simulink model "pasp_16i16c1ks"  (Simulink 7.3)
settings: Solver=ode45  StartTime=0.0  StopTime=10  RelTol=1e-3
undefined symbols (not defined in the file or in its folder): BEE2_ctrl, dist_gbe, ip_ctr, makereorderarray, pasp_lib, pfb_coeff_gen_calc
libraries: xbsIndex_r4 x2297, casper_library x261, xps_library x57, gavrt_library x2
(part 5 of 28)

== system pasp_16i16c1ks/pasp/scale_ctr ==
  Reset : Inport  OutDataType=sfix(16)  OutScaling=2^0
  Counter : xbsIndex_r4/Counter  cnt_type=Free Running  cnt_to=Inf  operation=Up  start_count=0  cnt_by_val=1  arith_type=Unsigned  n_bits=3  bin_pt=0  load_pin=off  rst=on  en=off  explicit_period=off  period=1  dbl_ovrd=off  use_behavioral_HDL=off  implementation=Fabric  use_rpm=on  block_type=counter
      settings: xl_area=[0, 0, 0, 0, 0, 0, 0]
  data_in : xbsIndex_r4/Constant  arith_type=Unsigned  const=1  n_bits=32  bin_pt=0  explicit_period=on  period=1  equ=P=C  opselect=C  inp2=PCIN>>17  opr=+  inp1=P  carry=CIN  dbl_ovrd=off  block_type=constant
  pol0_even_bram : xps_library/Shared BRAM  Tag=xps:bram  SystemSampleTime=-1  arith_type=Unsigned  addr_width=11  data_width=32  data_bin_pt=0  init_vals=1:8  sample_rate=1
      link override convert_din: arith_type=Unsigned
      link override mem/sim_data_in: arith_type=Unsigned
      link override pasp_16i16c128s_pasp_scale_ctr_pol0_even_bram_data_out: arith_type=Unsigned
  pol0_odd_bram : xps_library/Shared BRAM  Tag=xps:bram  SystemSampleTime=-1  arith_type=Unsigned  addr_width=11  data_width=32  data_bin_pt=0  init_vals=1:8  sample_rate=1
      link override convert_din: arith_type=Unsigned
      link override mem/sim_data_in: arith_type=Unsigned
      link override pasp_16i16c128s_pasp_scale_ctr_pol0_odd_bram_data_out: arith_type=Unsigned
  pol1_even_bram : xps_library/Shared BRAM  Tag=xps:bram  SystemSampleTime=-1  arith_type=Unsigned  addr_width=11  data_width=32  data_bin_pt=0  init_vals=1:8  sample_rate=1
      link override convert_din: arith_type=Unsigned
      link override mem/sim_data_in: arith_type=Unsigned
      link override pasp_16i16c128s_pasp_scale_ctr_pol1_even_bram_data_out: arith_type=Unsigned
  pol1_odd_bram : xps_library/Shared BRAM  Tag=xps:bram  SystemSampleTime=-1  arith_type=Unsigned  addr_width=11  data_width=32  data_bin_pt=0  init_vals=1:8  sample_rate=1
      link override convert_din: arith_type=Unsigned
      link override mem/sim_data_in: arith_type=Unsigned
      link override pasp_16i16c128s_pasp_scale_ctr_pol1_odd_bram_data_out: arith_type=Unsigned
  we : xbsIndex_r4/Constant  arith_type=Boolean  const=0  n_bits=1  bin_pt=0  explicit_period=on  period=1  equ=P=C  opselect=C  inp2=PCIN>>17  opr=+  inp1=P  carry=CIN  dbl_ovrd=off  block_type=constant
  Pol0_Odd : Outport  OutDataType=sfix(16)  OutScaling=2^0
  Pol0_Even : Outport  Port=2  OutDataType=sfix(16)  OutScaling=2^0
  Pol1_Odd : Outport  Port=3  OutDataType=sfix(16)  OutScaling=2^0
  Pol1_Even : Outport  Port=4  OutDataType=sfix(16)  OutScaling=2^0
  wires:
  Reset/1 -> Counter/1
  Counter/1 -> pol0_odd_bram/1, pol0_even_bram/1, pol1_odd_bram/1, pol1_even_bram/1
  data_in/1 -> pol0_odd_bram/2, pol0_even_bram/2, pol1_odd_bram/2, pol1_even_bram/2
  we/1 -> pol0_odd_bram/3, pol0_even_bram/3, pol1_odd_bram/3, pol1_even_bram/3
  pol0_odd_bram/1 -> Pol0_Odd/1
  pol0_even_bram/1 -> Pol0_Even/1
  pol1_odd_bram/1 -> Pol1_Odd/1
  pol1_even_bram/1 -> Pol1_Even/1

== system pasp_16i16c1ks/pasp/scope_output1 ==
  rst : Inport  OutDataType=sfix(16)  OutScaling=2^0
  en : Inport  Port=2  OutDataType=sfix(16)  OutScaling=2^0
  data_in : Inport  Port=3  OutDataType=sfix(16)  OutScaling=2^0
  bram : xps_library/Shared BRAM  Tag=xps:bram  SystemSampleTime=-1  arith_type="Signed  (2's comp)"  addr_width=11  data_width=32  data_bin_pt=17  init_vals=[1:2^11]  sample_rate=1
  freeze_cntr : casper_library/Misc/freeze_cntr  SystemSampleTime=-1  CounterBits=11
  wires:
  freeze_cntr/1 -> bram/1
  freeze_cntr/2 -> bram/3
  en/1 -> freeze_cntr/1
  data_in/1 -> bram/2
  rst/1 -> freeze_cntr/2

== system pasp_16i16c1ks/pasp/scope_output2 ==
  rst : Inport  OutDataType=sfix(16)  OutScaling=2^0
  en : Inport  Port=2  OutDataType=sfix(16)  OutScaling=2^0
  data_in : Inport  Port=3  OutDataType=sfix(16)  OutScaling=2^0
  bram : xps_library/Shared BRAM  Tag=xps:bram  SystemSampleTime=-1  arith_type="Signed  (2's comp)"  addr_width=11  data_width=32  data_bin_pt=17  init_vals=[1:2^11]  sample_rate=1
  freeze_cntr : casper_library/Misc/freeze_cntr  SystemSampleTime=-1  CounterBits=11
  wires:
  freeze_cntr/1 -> bram/1
  freeze_cntr/2 -> bram/3
  en/1 -> freeze_cntr/1
  data_in/1 -> bram/2
  rst/1 -> freeze_cntr/2

== system pasp_16i16c1ks/pasp/scope_output3 ==
  rst : Inport  OutDataType=sfix(16)  OutScaling=2^0
  en : Inport  Port=2  OutDataType=sfix(16)  OutScaling=2^0
  data_in : Inport  Port=3  OutDataType=sfix(16)  OutScaling=2^0
  bram : xps_library/Shared BRAM  Tag=xps:bram  SystemSampleTime=-1  arith_type="Signed  (2's comp)"  addr_width=11  data_width=32  data_bin_pt=17  init_vals=[1:2^11]  sample_rate=1
  freeze_cntr : casper_library/Misc/freeze_cntr  SystemSampleTime=-1  CounterBits=11
  wires:
  rst/1 -> freeze_cntr/2
  data_in/1 -> bram/2
  en/1 -> freeze_cntr/1
  freeze_cntr/2 -> bram/3
  freeze_cntr/1 -> bram/1

== system pasp_16i16c1ks/pasp/scope_output4 ==
  rst : Inport  OutDataType=sfix(16)  OutScaling=2^0
  en : Inport  Port=2  OutDataType=sfix(16)  OutScaling=2^0
  data_in : Inport  Port=3  OutDataType=sfix(16)  OutScaling=2^0
  bram : xps_library/Shared BRAM  Tag=xps:bram  SystemSampleTime=-1  arith_type="Signed  (2's comp)"  addr_width=11  data_width=32  data_bin_pt=17  init_vals=[1:2^11]  sample_rate=1
  freeze_cntr : casper_library/Misc/freeze_cntr  SystemSampleTime=-1  CounterBits=11
  wires:
  freeze_cntr/1 -> bram/1
  freeze_cntr/2 -> bram/3
  en/1 -> freeze_cntr/1
  data_in/1 -> bram/2
  rst/1 -> freeze_cntr/2

== system pasp_16i16c1ks/pasp/scope_output5 ==
  rst : Inport  OutDataType=sfix(16)  OutScaling=2^0
  en : Inport  Port=2  OutDataType=sfix(16)  OutScaling=2^0
  data_in : Inport  Port=3  OutDataType=sfix(16)  OutScaling=2^0
  bram : xps_library/Shared BRAM  Tag=xps:bram  SystemSampleTime=-1  arith_type="Signed  (2's comp)"  addr_width=11  data_width=32  data_bin_pt=17  init_vals=[1:2^11]  sample_rate=1
  freeze_cntr : casper_library/Misc/freeze_cntr  SystemSampleTime=-1  CounterBits=11
  wires:
  freeze_cntr/1 -> bram/1
  freeze_cntr/2 -> bram/3
  en/1 -> freeze_cntr/1
  data_in/1 -> bram/2
  rst/1 -> freeze_cntr/2

== system pasp_16i16c1ks/pasp/scope_output6 ==
  rst : Inport  OutDataType=sfix(16)  OutScaling=2^0
  en : Inport  Port=2  OutDataType=sfix(16)  OutScaling=2^0
  data_in : Inport  Port=3  OutDataType=sfix(16)  OutScaling=2^0
  bram : xps_library/Shared BRAM  Tag=xps:bram  SystemSampleTime=-1  arith_type="Signed  (2's comp)"  addr_width=11  data_width=32  data_bin_pt=17  init_vals=[1:2^11]  sample_rate=1
  freeze_cntr : casper_library/Misc/freeze_cntr  SystemSampleTime=-1  CounterBits=11
  wires:
  freeze_cntr/1 -> bram/1
  freeze_cntr/2 -> bram/3
  en/1 -> freeze_cntr/1
  data_in/1 -> bram/2
  rst/1 -> freeze_cntr/2

== system pasp_16i16c1ks/pasp/scope_output7 ==
  rst : Inport  OutDataType=sfix(16)  OutScaling=2^0
  en : Inport  Port=2  OutDataType=sfix(16)  OutScaling=2^0
  data_in : Inport  Port=3  OutDataType=sfix(16)  OutScaling=2^0
  bram : xps_library/Shared BRAM  Tag=xps:bram  SystemSampleTime=-1  arith_type="Signed  (2's comp)"  addr_width=11  data_width=32  data_bin_pt=17  init_vals=[1:2^11]  sample_rate=1
  freeze_cntr : casper_library/Misc/freeze_cntr  SystemSampleTime=-1  CounterBits=11
  wires:
  rst/1 -> freeze_cntr/2
  data_in/1 -> bram/2
  en/1 -> freeze_cntr/1
  freeze_cntr/2 -> bram/3
  freeze_cntr/1 -> bram/1

== system pasp_16i16c1ks/pasp/scope_output8 ==
  rst : Inport  OutDataType=sfix(16)  OutScaling=2^0
  en : Inport  Port=2  OutDataType=sfix(16)  OutScaling=2^0
  data_in : Inport  Port=3  OutDataType=sfix(16)  OutScaling=2^0
  bram : xps_library/Shared BRAM  Tag=xps:bram  SystemSampleTime=-1  arith_type="Signed  (2's comp)"  addr_width=11  data_width=32  data_bin_pt=17  init_vals=[1:2^11]  sample_rate=1
  freeze_cntr : casper_library/Misc/freeze_cntr  SystemSampleTime=-1  CounterBits=11
  wires:
  freeze_cntr/1 -> bram/1
  freeze_cntr/2 -> bram/3
  en/1 -> freeze_cntr/1
  data_in/1 -> bram/2
  rst/1 -> freeze_cntr/2

== system pasp_16i16c1ks/pasp/sync_generator ==
  period : Inport  OutDataType=sfix(16)  OutScaling=2^0
  reset : Inport  Port=2  OutDataType=sfix(16)  OutScaling=2^0
  Convert : xbsIndex_r4/Convert  arith_type=Boolean  n_bits=8  bin_pt=6  quantization=Truncate  overflow=Wrap  latency=1  dbl_ovrd=off  pipeline=off  block_type=convert
      settings: xl_area=[0, 0, 0, 0, 0, 0, 0]  block_version=10.1.3
  Counter : xbsIndex_r4/Counter  cnt_type=Free Running  cnt_to=Inf  operation=Up  start_count=1  cnt_by_val=1  arith_type=Unsigned  n_bits=32  bin_pt=0  load_pin=off  rst=on  en=off  explicit_period=on  period=1  dbl_ovrd=off  use_behavioral_HDL=off  implementation=Fabric  use_rpm=off  block_type=counter
  Logical : xbsIndex_r4/Logical  logical_function=OR  inputs=2  en=off  latency=0  precision=Full  arith_type=Unsigned  n_bits=8  bin_pt=2  align_bp=on  dbl_ovrd=off  block_type=logical
      settings: xl_area=[0, 0, 0, 0, 0, 0, 0]
  Relational : xbsIndex_r4/Relational  mode=a>=b  en=off  latency=1  dbl_ovrd=off  block_type=relational
      settings: xl_area=[0, 0, 0, 0, 0, 0, 0]  block_version=10.1.3
  cns : xbsIndex_r4/Constant  arith_type=Unsigned  const=4  n_bits=3  bin_pt=0  explicit_period=off  period=1  equ=P=C  opselect=C  inp2=PCIN>>17  opr=+  inp1=P  carry=CIN  dbl_ovrd=off  block_type=constant
  period_sub : xbsIndex_r4/AddSub  mode=Subtraction  use_carryin=off  use_carryout=off  en=off  latency=0  precision=User Defined  arith_type=Unsigned  n_bits=32  bin_pt=0  quantization=Truncate  overflow=Wrap  dbl_ovrd=off  use_behavioral_HDL=on  hw_selection=Fabric  pipelined=off  use_rpm=on  block_type=addsub
      settings: xl_area=[0, 0, 0, 0, 0, 0, 0]  block_version=10.1.3
  posedge : casper_library/Misc/posedge  Tag=Rising Edge Detector  SystemSampleTime=-1
  sync : Outport  OutDataType=sfix(16)  OutScaling=2^0
  wires:
  Counter/1 -> Relational/1
  Relational/1 -> Convert/1
  Convert/1 -> Logical/2
  Logical/1 -> posedge/1, Counter/1
  reset/1 -> Logical/1
  period/1 -> period_sub/1
  period_sub/1 -> Relational/2
  cns/1 -> period_sub/2
  posedge/1 -> sync/1

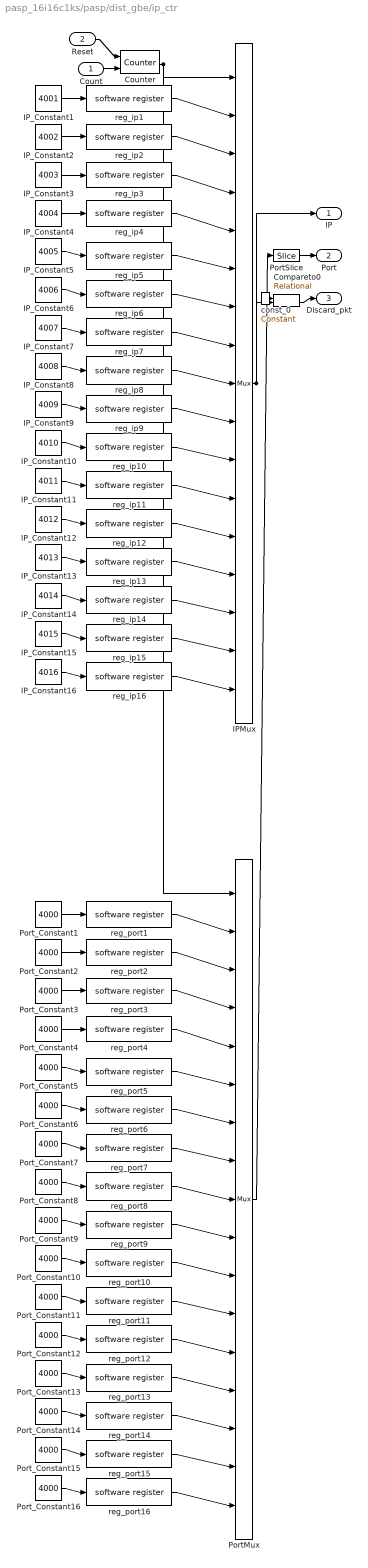
== system pasp_16i16c1ks/pasp/dist_gbe/ip_ctr ==
  Count : Inport
  Reset : Inport  Port=2  OutDataType=sfix(16)  OutScaling=2^0
  Compareto0 : xbsIndex_r4/Relational  mode=a=b  en=off  latency=1  dbl_ovrd=off  block_type=relational
  Counter : xbsIndex_r4/Counter  cnt_type=Free Running  cnt_to=Inf  operation=Up  start_count=0  cnt_by_val=1  arith_type=Unsigned  n_bits=4  bin_pt=0  load_pin=off  rst=on  en=on  explicit_period=on  period=1  dbl_ovrd=off  use_behavioral_HDL=off  implementation=Fabric  block_type=counter
      settings: block_version=11.4
  IPMux : xbsIndex_r4/Mux  inputs=16  en=off  latency=3  precision=Full  arith_type=Unsigned  n_bits=16  bin_pt=14  quantization=Truncate  overflow=Wrap  dbl_ovrd=off  block_type=mux
      settings: block_version=11.4
  IP_Constant1 : Constant  Value=4001  OutDataType=sfix(16)  OutScaling=2^0
  IP_Constant10 : Constant  Value=4010  OutDataType=fixdt(1, 16)  OutScaling=2^0
  IP_Constant11 : Constant  Value=4011  OutDataType=fixdt(1, 16)  OutScaling=2^0
  IP_Constant12 : Constant  Value=4012  OutDataType=fixdt(1, 16)  OutScaling=2^0
  IP_Constant13 : Constant  Value=4013  OutDataType=fixdt(1, 16)  OutScaling=2^0
  IP_Constant14 : Constant  Value=4014  OutDataType=fixdt(1, 16)  OutScaling=2^0
  IP_Constant15 : Constant  Value=4015  OutDataType=fixdt(1, 16)  OutScaling=2^0
  IP_Constant16 : Constant  Value=4016  OutDataType=fixdt(1, 16)  OutScaling=2^0
  IP_Constant2 : Constant  Value=4002  OutDataType=sfix(16)  OutScaling=2^0
  IP_Constant3 : Constant  Value=4003  OutDataType=sfix(16)  OutScaling=2^0
  IP_Constant4 : Constant  Value=4004  OutDataType=fixdt(1, 16)  OutScaling=2^0
  IP_Constant5 : Constant  Value=4005  OutDataType=fixdt(1, 16)  OutScaling=2^0
  IP_Constant6 : Constant  Value=4006  OutDataType=fixdt(1, 16)  OutScaling=2^0
  IP_Constant7 : Constant  Value=4007  OutDataType=fixdt(1, 16)  OutScaling=2^0
  IP_Constant8 : Constant  Value=4008  OutDataType=fixdt(1, 16)  OutScaling=2^0
  IP_Constant9 : Constant  Value=4009  OutDataType=fixdt(1, 16)  OutScaling=2^0
  PortMux : xbsIndex_r4/Mux  inputs=16  en=off  latency=3  precision=Full  arith_type=Unsigned  n_bits=16  bin_pt=14  quantization=Truncate  overflow=Wrap  dbl_ovrd=off  block_type=mux
      settings: block_version=11.4
  PortSlice : xbsIndex_r4/Slice  nbits=16  boolean_output=off  mode=Lower Bit Location + Width  bit1=0  base1=MSB of Input  bit0=0  base0=LSB of Input  dbl_ovrd=off  block_type=slice
  Port_Constant1 : Constant  Value=4000  OutDataType=sfix(16)  OutScaling=2^0
  Port_Constant{4..16} (13 alike) : Constant  Value=4000  OutDataType=fixdt(1, 16)  OutScaling=2^0
  Port_Constant2 : Constant  Value=4000  OutDataType=sfix(16)  OutScaling=2^0
  Port_Constant3 : Constant  Value=4000  OutDataType=sfix(16)  OutScaling=2^0
  const_0 : xbsIndex_r4/Constant  arith_type=Unsigned  const=0  n_bits=32  bin_pt=0  explicit_period=off  period=1  equ=P=C  opselect=C  inp2=PCIN>>17  opr=+  inp1=P  carry=CIN  dbl_ovrd=off  block_type=constant
      settings: block_version=11.4
  reg_ip1 : xps_library/software register  Tag=xps:sw_reg  SystemSampleTime=-1  io_dir=From Processor  arith_type=Unsigned  bitwidth=32  bin_pt=0  sample_period=1  gw_name=pasp_16i16c1ks_pasp_dist_gbe_ip_ctr_reg_ip1_user_data_out
      link override pasp_16i16c128s_pasp_dist_gbe_ip_ctr_reg_ip1_user_data_out: arith_type=Unsigned  n_bits=32  bin_pt=0  period=1
  reg_ip10 : xps_library/software register  Tag=xps:sw_reg  SystemSampleTime=-1  io_dir=From Processor  arith_type=Unsigned  bitwidth=32  bin_pt=0  sample_period=1  gw_name=pasp_16i16c1ks_pasp_dist_gbe_ip_ctr_reg_ip10_user_data_out
  reg_ip11 : xps_library/software register  Tag=xps:sw_reg  SystemSampleTime=-1  io_dir=From Processor  arith_type=Unsigned  bitwidth=32  bin_pt=0  sample_period=1  gw_name=pasp_16i16c1ks_pasp_dist_gbe_ip_ctr_reg_ip11_user_data_out
  reg_ip12 : xps_library/software register  Tag=xps:sw_reg  SystemSampleTime=-1  io_dir=From Processor  arith_type=Unsigned  bitwidth=32  bin_pt=0  sample_period=1  gw_name=pasp_16i16c1ks_pasp_dist_gbe_ip_ctr_reg_ip12_user_data_out
  reg_ip13 : xps_library/software register  Tag=xps:sw_reg  SystemSampleTime=-1  io_dir=From Processor  arith_type=Unsigned  bitwidth=32  bin_pt=0  sample_period=1  gw_name=pasp_16i16c1ks_pasp_dist_gbe_ip_ctr_reg_ip13_user_data_out
  reg_ip14 : xps_library/software register  Tag=xps:sw_reg  SystemSampleTime=-1  io_dir=From Processor  arith_type=Unsigned  bitwidth=32  bin_pt=0  sample_period=1  gw_name=pasp_16i16c1ks_pasp_dist_gbe_ip_ctr_reg_ip14_user_data_out
  reg_ip15 : xps_library/software register  Tag=xps:sw_reg  SystemSampleTime=-1  io_dir=From Processor  arith_type=Unsigned  bitwidth=32  bin_pt=0  sample_period=1  gw_name=pasp_16i16c1ks_pasp_dist_gbe_ip_ctr_reg_ip15_user_data_out
  reg_ip16 : xps_library/software register  Tag=xps:sw_reg  SystemSampleTime=-1  io_dir=From Processor  arith_type=Unsigned  bitwidth=32  bin_pt=0  sample_period=1  gw_name=pasp_16i16c1ks_pasp_dist_gbe_ip_ctr_reg_ip16_user_data_out
  reg_ip2 : xps_library/software register  Tag=xps:sw_reg  SystemSampleTime=-1  io_dir=From Processor  arith_type=Unsigned  bitwidth=32  bin_pt=0  sample_period=1  gw_name=pasp_16i16c1ks_pasp_dist_gbe_ip_ctr_reg_ip2_user_data_out
      link override pasp_16i16c128s_pasp_dist_gbe_ip_ctr_reg_ip2_user_data_out: arith_type=Unsigned  n_bits=32  bin_pt=0  period=1
  reg_ip3 : xps_library/software register  Tag=xps:sw_reg  SystemSampleTime=-1  io_dir=From Processor  arith_type=Unsigned  bitwidth=32  bin_pt=0  sample_period=1  gw_name=pasp_16i16c1ks_pasp_dist_gbe_ip_ctr_reg_ip3_user_data_out
      link override pasp_16i16c128s_pasp_dist_gbe_ip_ctr_reg_ip3_user_data_out: arith_type=Unsigned  n_bits=32  bin_pt=0  period=1
  reg_ip4 : xps_library/software register  Tag=xps:sw_reg  SystemSampleTime=-1  io_dir=From Processor  arith_type=Unsigned  bitwidth=32  bin_pt=0  sample_period=1  gw_name=pasp_16i16c1ks_pasp_dist_gbe_ip_ctr_reg_ip4_user_data_out
  reg_ip5 : xps_library/software register  Tag=xps:sw_reg  SystemSampleTime=-1  io_dir=From Processor  arith_type=Unsigned  bitwidth=32  bin_pt=0  sample_period=1  gw_name=pasp_16i16c1ks_pasp_dist_gbe_ip_ctr_reg_ip5_user_data_out
  reg_ip6 : xps_library/software register  Tag=xps:sw_reg  SystemSampleTime=-1  io_dir=From Processor  arith_type=Unsigned  bitwidth=32  bin_pt=0  sample_period=1  gw_name=pasp_16i16c1ks_pasp_dist_gbe_ip_ctr_reg_ip6_user_data_out
  reg_ip7 : xps_library/software register  Tag=xps:sw_reg  SystemSampleTime=-1  io_dir=From Processor  arith_type=Unsigned  bitwidth=32  bin_pt=0  sample_period=1  gw_name=pasp_16i16c1ks_pasp_dist_gbe_ip_ctr_reg_ip7_user_data_out
  reg_ip8 : xps_library/software register  Tag=xps:sw_reg  SystemSampleTime=-1  io_dir=From Processor  arith_type=Unsigned  bitwidth=32  bin_pt=0  sample_period=1  gw_name=pasp_16i16c1ks_pasp_dist_gbe_ip_ctr_reg_ip8_user_data_out
  reg_ip9 : xps_library/software register  Tag=xps:sw_reg  SystemSampleTime=-1  io_dir=From Processor  arith_type=Unsigned  bitwidth=32  bin_pt=0  sample_period=1  gw_name=pasp_16i16c1ks_pasp_dist_gbe_ip_ctr_reg_ip9_user_data_out
  reg_port1 : xps_library/software register  Tag=xps:sw_reg  SystemSampleTime=-1  io_dir=From Processor  arith_type=Unsigned  bitwidth=32  bin_pt=0  sample_period=1  gw_name=pasp_16i16c1ks_pasp_dist_gbe_ip_ctr_reg_port1_user_data_out
      link override pasp_16i16c128s_pasp_dist_gbe_ip_ctr_reg_port1_user_data_out: arith_type=Unsigned  n_bits=32  bin_pt=0  period=1
  reg_port10 : xps_library/software register  Tag=xps:sw_reg  SystemSampleTime=-1  io_dir=From Processor  arith_type=Unsigned  bitwidth=32  bin_pt=0  sample_period=1  gw_name=pasp_16i16c1ks_pasp_dist_gbe_ip_ctr_reg_port10_user_data_out
  reg_port11 : xps_library/software register  Tag=xps:sw_reg  SystemSampleTime=-1  io_dir=From Processor  arith_type=Unsigned  bitwidth=32  bin_pt=0  sample_period=1  gw_name=pasp_16i16c1ks_pasp_dist_gbe_ip_ctr_reg_port11_user_data_out
  reg_port12 : xps_library/software register  Tag=xps:sw_reg  SystemSampleTime=-1  io_dir=From Processor  arith_type=Unsigned  bitwidth=32  bin_pt=0  sample_period=1  gw_name=pasp_16i16c1ks_pasp_dist_gbe_ip_ctr_reg_port12_user_data_out
  reg_port13 : xps_library/software register  Tag=xps:sw_reg  SystemSampleTime=-1  io_dir=From Processor  arith_type=Unsigned  bitwidth=32  bin_pt=0  sample_period=1  gw_name=pasp_16i16c1ks_pasp_dist_gbe_ip_ctr_reg_port13_user_data_out
  reg_port14 : xps_library/software register  Tag=xps:sw_reg  SystemSampleTime=-1  io_dir=From Processor  arith_type=Unsigned  bitwidth=32  bin_pt=0  sample_period=1  gw_name=pasp_16i16c1ks_pasp_dist_gbe_ip_ctr_reg_port14_user_data_out
  reg_port15 : xps_library/software register  Tag=xps:sw_reg  SystemSampleTime=-1  io_dir=From Processor  arith_type=Unsigned  bitwidth=32  bin_pt=0  sample_period=1  gw_name=pasp_16i16c1ks_pasp_dist_gbe_ip_ctr_reg_port15_user_data_out
  reg_port16 : xps_library/software register  Tag=xps:sw_reg  SystemSampleTime=-1  io_dir=From Processor  arith_type=Unsigned  bitwidth=32  bin_pt=0  sample_period=1  gw_name=pasp_16i16c1ks_pasp_dist_gbe_ip_ctr_reg_port16_user_data_out
  reg_port2 : xps_library/software register  Tag=xps:sw_reg  SystemSampleTime=-1  io_dir=From Processor  arith_type=Unsigned  bitwidth=32  bin_pt=0  sample_period=1  gw_name=pasp_16i16c1ks_pasp_dist_gbe_ip_ctr_reg_port2_user_data_out
      link override pasp_16i16c128s_pasp_dist_gbe_ip_ctr_reg_port2_user_data_out: arith_type=Unsigned  n_bits=32  bin_pt=0  period=1
  reg_port3 : xps_library/software register  Tag=xps:sw_reg  SystemSampleTime=-1  io_dir=From Processor  arith_type=Unsigned  bitwidth=32  bin_pt=0  sample_period=1  gw_name=pasp_16i16c1ks_pasp_dist_gbe_ip_ctr_reg_port3_user_data_out
      link override pasp_16i16c128s_pasp_dist_gbe_ip_ctr_reg_port3_user_data_out: arith_type=Unsigned  n_bits=32  bin_pt=0  period=1
  reg_port4 : xps_library/software register  Tag=xps:sw_reg  SystemSampleTime=-1  io_dir=From Processor  arith_type=Unsigned  bitwidth=32  bin_pt=0  sample_period=1  gw_name=pasp_16i16c1ks_pasp_dist_gbe_ip_ctr_reg_port4_user_data_out
  reg_port5 : xps_library/software register  Tag=xps:sw_reg  SystemSampleTime=-1  io_dir=From Processor  arith_type=Unsigned  bitwidth=32  bin_pt=0  sample_period=1  gw_name=pasp_16i16c1ks_pasp_dist_gbe_ip_ctr_reg_port5_user_data_out
  reg_port6 : xps_library/software register  Tag=xps:sw_reg  SystemSampleTime=-1  io_dir=From Processor  arith_type=Unsigned  bitwidth=32  bin_pt=0  sample_period=1  gw_name=pasp_16i16c1ks_pasp_dist_gbe_ip_ctr_reg_port6_user_data_out
  reg_port7 : xps_library/software register  Tag=xps:sw_reg  SystemSampleTime=-1  io_dir=From Processor  arith_type=Unsigned  bitwidth=32  bin_pt=0  sample_period=1  gw_name=pasp_16i16c1ks_pasp_dist_gbe_ip_ctr_reg_port7_user_data_out
  reg_port8 : xps_library/software register  Tag=xps:sw_reg  SystemSampleTime=-1  io_dir=From Processor  arith_type=Unsigned  bitwidth=32  bin_pt=0  sample_period=1  gw_name=pasp_16i16c1ks_pasp_dist_gbe_ip_ctr_reg_port8_user_data_out
  reg_port9 : xps_library/software register  Tag=xps:sw_reg  SystemSampleTime=-1  io_dir=From Processor  arith_type=Unsigned  bitwidth=32  bin_pt=0  sample_period=1  gw_name=pasp_16i16c1ks_pasp_dist_gbe_ip_ctr_reg_port9_user_data_out
  IP : Outport
  Port : Outport  Port=2
  Discard_pkt : Outport  Port=3
  wires:
  Reset/1 -> Counter/1
  Count/1 -> Counter/2
  Counter/1 -> IPMux/1, PortMux/1
  IPMux/1 -> IP/1, Compareto0/2
  PortMux/1 -> PortSlice/1
  PortSlice/1 -> Port/1
  const_0/1 -> Compareto0/1
  Compareto0/1 -> Discard_pkt/1
  IP_Constant1/1 -> reg_ip1/1
  reg_ip1/1 -> IPMux/2
  Port_Constant1/1 -> reg_port1/1
  reg_port1/1 -> PortMux/2
  IP_Constant2/1 -> reg_ip2/1
  reg_ip2/1 -> IPMux/3
  Port_Constant2/1 -> reg_port2/1
  reg_port2/1 -> PortMux/3
  IP_Constant3/1 -> reg_ip3/1
  reg_ip3/1 -> IPMux/4
  Port_Constant3/1 -> reg_port3/1
  reg_port3/1 -> PortMux/4
  IP_Constant4/1 -> reg_ip4/1
  reg_ip4/1 -> IPMux/5
  Port_Constant4/1 -> reg_port4/1
  reg_port4/1 -> PortMux/5
  IP_Constant5/1 -> reg_ip5/1
  reg_ip5/1 -> IPMux/6
  Port_Constant5/1 -> reg_port5/1
  reg_port5/1 -> PortMux/6
  IP_Constant6/1 -> reg_ip6/1
  reg_ip6/1 -> IPMux/7
  Port_Constant6/1 -> reg_port6/1
  reg_port6/1 -> PortMux/7
  IP_Constant7/1 -> reg_ip7/1
  reg_ip7/1 -> IPMux/8
  Port_Constant7/1 -> reg_port7/1
  reg_port7/1 -> PortMux/8
  IP_Constant8/1 -> reg_ip8/1
  reg_ip8/1 -> IPMux/9
  Port_Constant8/1 -> reg_port8/1
  reg_port8/1 -> PortMux/9
  IP_Constant9/1 -> reg_ip9/1
  reg_ip9/1 -> IPMux/10
  Port_Constant9/1 -> reg_port9/1
  reg_port9/1 -> PortMux/10
  IP_Constant10/1 -> reg_ip10/1
  reg_ip10/1 -> IPMux/11
  Port_Constant10/1 -> reg_port10/1
  reg_port10/1 -> PortMux/11
  IP_Constant11/1 -> reg_ip11/1
  reg_ip11/1 -> IPMux/12
  Port_Constant11/1 -> reg_port11/1
  reg_port11/1 -> PortMux/12
  IP_Constant12/1 -> reg_ip12/1
  reg_ip12/1 -> IPMux/13
  Port_Constant12/1 -> reg_port12/1
  reg_port12/1 -> PortMux/13
  IP_Constant13/1 -> reg_ip13/1
  reg_ip13/1 -> IPMux/14
  Port_Constant13/1 -> reg_port13/1
  reg_port13/1 -> PortMux/14
  IP_Constant14/1 -> reg_ip14/1
  reg_ip14/1 -> IPMux/15
  Port_Constant14/1 -> reg_port14/1
  reg_port14/1 -> PortMux/15
  IP_Constant15/1 -> reg_ip15/1
  reg_ip15/1 -> IPMux/16
  Port_Constant15/1 -> reg_port15/1
  reg_port15/1 -> PortMux/16
  IP_Constant16/1 -> reg_ip16/1
  reg_ip16/1 -> IPMux/17
  Port_Constant16/1 -> reg_port16/1
  reg_port16/1 -> PortMux/17
